simulink model "TosAeb"  (R2021a)
settings: Solver=FixedStepDiscrete  StartTime=0.0  StopTime=inf  FixedStep=0.01  RelTol=1e-3
undefined symbols (not defined in the file or in its folder): ACCModSts, AccSts1, AEB_ObjectMovingOrientation, AEB_ObjectMovingState, CN_BTCActive, CN_CurrentGearR, CN_CurrentGearR_DHT, CN_PTCActive, CN_VDCActive, CN_VSC_EspOff, CN_VSC_FCW_AEB_Error, CN_VSC_FCW_AEB_NoDispay, CN_VSC_FCW_AEB_PerfmDgdtn, CN_VSC_FCW_AEB_TempUnAvlbl, CN_VSC_VehStandstill, CNaccelAvoidMax, CNaccelAvoidMin, CNbrakePedalPress, CNcomfortStrLeftAccelMax, CNcomfortStrLeftAccelMin, CNcomfortStrRightAccelMax, CNcomfortStrRightAccelMin, CNDrvDoorOpen, CNDrvModeEco, CNDrvModeNorm, CNDrvModeOther, CNDrvModeSport, CNDrvSBRreminder, CNNull, CNobjInvalidID, CNPassDoorOpen, CNPassSBRreminder, CNPEBSAccelNegInf, CNPEBSAccelNull, CNPEBSTimeInf, CNPEBSTimeNull, CNSTOPMAX, CNSTOPNULL, CNsubjWidth, CNSwtOFF, CNSwtON, CNTTCMAX, CNTTCNULL, CNVehConfigAEBCycNone, CNVehConfigAEBNone, CNVehConfigAEBPedNone, CNVehConfigFCWNone, CNVehConfigJANone, CollisionSeverity, FALSE, FCWSnvty, JACycObjCalcInfoDefaultParams, JAPedObjCalcInfoDefaultParams, JAVehObjCalcInfoDefaultParams, latCycObjCalcInfoDefaultParams, latPedObjCalcInfoDefaultParams, longObjCalcInfoDefaultParams, ModSts, movingStatus, ObjectType ... (+1112)
libraries: simulink x3031, AEBSFunctionLib x50, Ta_Library x33, AEBSStateMachineLib x23, Mylibrary x12, simulink_extras x7, ObjSelect_lib x3, dspstat3 x2
(part 38 of 102)

== system TosAeb/FcwAebJa_Step_sys/ALG_AEBSystem/ALG_AEBController/AEB_FuncCondCalcApp/CycFunctionCondCalc/cycPrefillSuppAborCond/cycPrefillSuppCondCalc/cycPrefillSuppCondFinalJudge ==
  cycPrefillObjRelSuppCondFulfilled : Inport
  cycPrefillVehRelSuppCondFulfilled : Inport  Port=2
  cycPrefillSituationRelSuppCondFulfilled : Inport  Port=3
  Compare To Constant : simulink/Logic and Bit Operations/Compare To Constant  relop===  const=TRUE  OutDataTypeStr=boolean
  Compare To Constant1 : simulink/Logic and Bit Operations/Compare To Constant  relop===  const=TRUE  OutDataTypeStr=boolean
  Compare To Constant2 : simulink/Logic and Bit Operations/Compare To Constant  relop===  const=TRUE  OutDataTypeStr=boolean
  Logical Operator : Logic  Operator=OR  Inputs=3  AllPortsSameDT=off  OutDataTypeStr=boolean
  cycPrefillSuppCondFulfilled : Outport
  wires:
  Logical Operator/1 -> cycPrefillSuppCondFulfilled/1
  cycPrefillSituationRelSuppCondFulfilled/1 -> Compare To Constant2/1
  cycPrefillObjRelSuppCondFulfilled/1 -> Compare To Constant/1
  cycPrefillVehRelSuppCondFulfilled/1 -> Compare To Constant1/1
  Compare To Constant/1 -> Logical Operator/1
  Compare To Constant1/1 -> Logical Operator/2
  Compare To Constant2/1 -> Logical Operator/3

== system TosAeb/FcwAebJa_Step_sys/ALG_AEBSystem/ALG_AEBController/AEB_FuncCondCalcApp/CycFunctionCondCalc/cycPrefillSuppAborCond/cycPrefillSuppCondCalc/cycPrefillVehRelSuppCondCalc ==
  subjVel : Inport
  VehConfigIbooster : Inport  Port=2
  ESP_MasterCylindBrakePressValid : Inport  Port=3
  ESP_MasterCylindBrakePress : Inport  Port=4
  IB_BrkPedalAppld : Inport  Port=5
  SteerWheelAngle : Inport  Port=6
  SteerWheelSpd : Inport  Port=7
  AccrCyclPosnDiagc : Inport  Port=8
  vehStateCalcCycPrefillSuppFlg : Inport  Port=9
  Abs : Abs
  Abs1 : Abs
  AccReleventSuppCondDelayAbortion : AEBSFunctionLib/StateDelayAbortion  SystemSampleTime=-1  VariantActivationTime=update diagram  Latency=0  AutoFrameSizeCalculation=off
  Compare To Constant : simulink/Logic and Bit Operations/Compare To Constant  relop=>  const=P_AEB_Func_cycPrefillStrWheelAngleSupThres  OutDataTypeStr=boolean
  Compare To Constant1 : simulink/Logic and Bit Operations/Compare To Constant  relop=>  const=P_AEB_Func_cycPrefillGasPedalSupThres  OutDataTypeStr=boolean
  Compare To Constant2 : simulink/Logic and Bit Operations/Compare To Constant  relop=>  const=P_AEB_Func_cycPrefillStrWheelAngleSpdSupThres  OutDataTypeStr=boolean
  Compare To Constant7 : simulink/Logic and Bit Operations/Compare To Constant  relop===  const=TRUE  OutDataTypeStr=boolean
  Compare To Constant8 : simulink/Logic and Bit Operations/Compare To Constant  relop=<  const=P_AEB_Func_cycPrefillSubjVelMin  OutDataTypeStr=boolean
  Compare To Constant9 : simulink/Logic and Bit Operations/Compare To Constant  relop=>  const=P_AEB_Func_cycPrefillSubjVelMax  OutDataTypeStr=boolean
  Constant : Constant  Value=P_AEB_Func_cycPrefillStrCondAborDelayTime
  Constant1 : Constant  Value=0.02
  Constant2 : Constant  Value=P_AEB_Func_cycPrefillAccrCondAborDelayTime
  Constant3 : Constant  Value=0.02
  Logical Operator : Logic  Operator=OR  AllPortsSameDT=off  OutDataTypeStr=boolean
  Logical Operator4 : Logic  Operator=OR  AllPortsSameDT=off  OutDataTypeStr=boolean
  Logical Operator5 : Logic  Operator=OR  Inputs=5  AllPortsSameDT=off  OutDataTypeStr=boolean
  StrReleventSuppCondDelayAbortion : AEBSFunctionLib/StateDelayAbortion  SystemSampleTime=-1  VariantActivationTime=update diagram  Latency=0  AutoFrameSizeCalculation=off
  brakeReleventSuppCond : Subsystem
  cycPrefillVehRelSuppCondFulfilled : Outport
  wires:
  IB_BrkPedalAppld/1 -> brakeReleventSuppCond/4(IB_BrkPedalAppld)
  ESP_MasterCylindBrakePress/1 -> brakeReleventSuppCond/3(ESP_MasterCylindBrakePress)
  ESP_MasterCylindBrakePressValid/1 -> brakeReleventSuppCond/2(ESP_MasterCylindBrakePressValid)
  VehConfigIbooster/1 -> brakeReleventSuppCond/1(VehConfigIbooster)
  Compare To Constant1/1 -> AccReleventSuppCondDelayAbortion/1
  Constant2/1 -> AccReleventSuppCondDelayAbortion/2
  Constant3/1 -> AccReleventSuppCondDelayAbortion/3
  AccrCyclPosnDiagc/1 -> Compare To Constant1/1
  Logical Operator4/1 -> StrReleventSuppCondDelayAbortion/1
  Constant1/1 -> StrReleventSuppCondDelayAbortion/3
  Constant/1 -> StrReleventSuppCondDelayAbortion/2
  Logical Operator5/1 -> cycPrefillVehRelSuppCondFulfilled/1  "cycPrefillVehRelSuppCondFulfilled"
  subjVel/1 -> Compare To Constant9/1, Compare To Constant8/1
  Logical Operator/1 -> Logical Operator5/1  "subjVelReleventSuppCondFulfilled"
  Compare To Constant7/1 -> Logical Operator5/5  "vehStateCalcCycPrefillSuppCondFulfilled"
  Compare To Constant9/1 -> Logical Operator/2
  brakeReleventSuppCond/1(brakeReleventSuppCond) -> Logical Operator5/2  "BrkReleventSuppCondFulfilled"
  StrReleventSuppCondDelayAbortion/1 -> Logical Operator5/3  "strReleventSuppCondFulfilled"
  SteerWheelAngle/1 -> Abs/1
  Abs/1 -> Compare To Constant/1
  Compare To Constant/1 -> Logical Operator4/1
  SteerWheelSpd/1 -> Abs1/1
  Compare To Constant2/1 -> Logical Operator4/2
  Compare To Constant8/1 -> Logical Operator/1
  vehStateCalcCycPrefillSuppFlg/1 -> Compare To Constant7/1
  Abs1/1 -> Compare To Constant2/1
  AccReleventSuppCondDelayAbortion/1 -> Logical Operator5/4  "accReleventSuppCondFulfilled"

== system TosAeb/FcwAebJa_Step_sys/ALG_AEBSystem/ALG_AEBController/AEB_FuncCondCalcApp/JaCycFunctionCondCalc/JaCycAEBfullSuppAborCond/JaCycAEBfullAborCondCalc/JaCycAEBfullAborCondFinalJudge ==
  JaCycAEBfullObjRelAborCond : Inport
  JaCycAEBfullVehRelAborCond : Inport  Port=2
  Compare To Constant1 : simulink/Logic and Bit Operations/Compare To Constant  relop===  const=TRUE  OutDataTypeStr=boolean
  Compare To Constant3 : simulink/Logic and Bit Operations/Compare To Constant  relop===  const=TRUE  OutDataTypeStr=boolean
  Logical Operator : Logic  Operator=OR  AllPortsSameDT=off  OutDataTypeStr=boolean
  JaCycAEBfullAborCondNoStndstillFulfilled : Outport
  JaCycAEBfullAborCondStndstillFulfilled : Outport  Port=2
  wires:
  Compare To Constant3/1 -> Logical Operator/1
  JaCycAEBfullVehRelAborCond/1 -> Compare To Constant1/1
  Logical Operator/1 -> JaCycAEBfullAborCondNoStndstillFulfilled/1  "AEBJAPedAEBfullAborCondNoStndstillFulfilled"
  JaCycAEBfullObjRelAborCond/1 -> Compare To Constant3/1
  Compare To Constant1/1 -> Logical Operator/2, JaCycAEBfullAborCondStndstillFulfilled/1  "AEBJAPedAEBfullAborCondStndstillFulfilled"

== system TosAeb/FcwAebJa_Step_sys/ALG_AEBSystem/ALG_AEBController/AEB_FuncCondCalcApp/JaCycFunctionCondCalc/JaCycAEBfullSuppAborCond/JaCycAEBfullAborCondCalc/JaCycAEBfullObjRelAborCondCalc ==
  ObjectID : Inport
  Compare To Constant4 : simulink/Logic and Bit Operations/Compare To Constant  relop===  const=CNobjInvalidID  OutDataTypeStr=boolean
  Logical Operator : Logic  Operator=OR  AllPortsSameDT=off  OutDataTypeStr=boolean
  Relational Operator2 : RelationalOperator  Operator=~=  OutDataTypeStr=boolean  RndMeth=Simplest
  Unit Delay1 : UnitDelay  SampleTime=-1  HasFrameUpgradeWarning=on
  JaCycAEBfullObjRelAborCond : Outport
  wires:
  Logical Operator/1 -> JaCycAEBfullObjRelAborCond/1
  ObjectID/1 -> Unit Delay1/1, Relational Operator2/1, Compare To Constant4/1
  Unit Delay1/1 -> Relational Operator2/2
  Compare To Constant4/1 -> Logical Operator/1
  Relational Operator2/1 -> Logical Operator/2

== system TosAeb/FcwAebJa_Step_sys/ALG_AEBSystem/ALG_AEBController/AEB_FuncCondCalcApp/JaCycFunctionCondCalc/JaCycAEBfullSuppAborCond/JaCycAEBfullAborCondCalc/JaCycAEBfullVehRelAborCondCalc ==
  SteerWheelSpd_DegS : Inport
  AccelPedalPositionDiag : Inport  Port=2
  VSC_JaCycAEBfullAborCond : Inport  Port=3
  Abs : Abs
  Abs1 : Abs
  Compare To Constant : simulink/Logic and Bit Operations/Compare To Constant  relop=>  const=P_AEB_Func_JaCycAEBfullStrWheelAngleSpdAborThres  OutDataTypeStr=boolean
  Compare To Constant1 : simulink/Logic and Bit Operations/Compare To Constant  relop=>  const=P_AEB_Func_JaCycAEBfullGasPedalAborThres  OutDataTypeStr=boolean
  Compare To Constant3 : simulink/Logic and Bit Operations/Compare To Constant  relop===  const=TRUE  OutDataTypeStr=boolean
  Logical Operator2 : Logic  Operator=OR  Inputs=3  AllPortsSameDT=off  OutDataTypeStr=boolean
  JaCycAEBfullVehRelAborCond : Outport
  wires:
  Compare To Constant3/1 -> Logical Operator2/3
  VSC_JaCycAEBfullAborCond/1 -> Compare To Constant3/1
  Logical Operator2/1 -> JaCycAEBfullVehRelAborCond/1  "AEBJAPedAEBfullVehRelAborCondFulfilled"
  SteerWheelSpd_DegS/1 -> Abs/1
  Abs1/1 -> Compare To Constant1/1
  AccelPedalPositionDiag/1 -> Abs1/1
  Abs/1 -> Compare To Constant/1
  Compare To Constant/1 -> Logical Operator2/1
  Compare To Constant1/1 -> Logical Operator2/2

== system TosAeb/FcwAebJa_Step_sys/ALG_AEBSystem/ALG_AEBController/AEB_FuncCondCalcApp/JaCycFunctionCondCalc/JaCycAEBfullSuppAborCond/JaCycAEBfullSuppCondCalc/JaCycAEBfullSuppCondFinalJudge ==
  JaCycAEBfullObjRelActiveCond : Inport
  JaCycAEBfullVehRelActiveCond : Inport  Port=2
  JaCycAEBfullSitRelActiveCond : Inport  Port=3
  Compare To Constant : simulink/Logic and Bit Operations/Compare To Constant  relop===  const=FALSE  OutDataTypeStr=boolean
  Compare To Constant1 : simulink/Logic and Bit Operations/Compare To Constant  relop===  const=FALSE  OutDataTypeStr=boolean
  Compare To Constant2 : simulink/Logic and Bit Operations/Compare To Constant  relop===  const=FALSE  OutDataTypeStr=boolean
  Logical Operator : Logic  Operator=OR  Inputs=3  AllPortsSameDT=off  OutDataTypeStr=boolean
  JaCycAEBfullSuppCond : Outport
  wires:
  Compare To Constant2/1 -> Logical Operator/3
  Compare To Constant1/1 -> Logical Operator/2
  Compare To Constant/1 -> Logical Operator/1
  JaCycAEBfullVehRelActiveCond/1 -> Compare To Constant1/1
  JaCycAEBfullObjRelActiveCond/1 -> Compare To Constant/1
  JaCycAEBfullSitRelActiveCond/1 -> Compare To Constant2/1
  Logical Operator/1 -> JaCycAEBfullSuppCond/1

== system TosAeb/FcwAebJa_Step_sys/ALG_AEBSystem/ALG_AEBController/AEB_FuncCondCalcApp/JaCycFunctionCondCalc/JaCycAEBfullSuppAborCond/JaCycAEBfullSuppCondCalc/JaCycAEBfullSuppObjCondCalc ==
  ObjectMovingOrientation : Inport
  ObjectID : Inport  Port=2
  ObjectExistProb : Inport  Port=3
  ObjectIsObstacleProb : Inport  Port=4
  ObjectTypeCameraConfirmed : Inport  Port=5
  dy : Inport  Port=6
  vxAbsObj : Inport  Port=7
  vyRelCorr : Inport  Port=8
  ObjFusQuality : Inport  Port=9
  Abs : Abs
  Compare To Constant1 : simulink/Logic and Bit Operations/Compare To Constant  relop=>  const=P_AEB_Func_JaCycAEBfullObjVelSupThresMin  OutDataTypeStr=boolean
  Compare To Constant11 : simulink/Logic and Bit Operations/Compare To Constant  relop===  const=TRUE  OutDataTypeStr=boolean
  Compare To Constant15 : simulink/Logic and Bit Operations/Compare To Constant  relop=~=  const=CNobjInvalidID  OutDataTypeStr=boolean
  Compare To Constant16 : simulink/Logic and Bit Operations/Compare To Constant  relop=>  const=P_AEB_Func_JaCycAEBfullObjObstacleProbSupThres  OutDataTypeStr=boolean
  Compare To Constant17 : simulink/Logic and Bit Operations/Compare To Constant  relop=~=  const=CNobjInvalidID  OutDataTypeStr=boolean
  Compare To Constant18 : simulink/Logic and Bit Operations/Compare To Constant  relop===  const=TRUE  OutDataTypeStr=boolean
  Compare To Constant2 : simulink/Logic and Bit Operations/Compare To Constant  relop=<  const=P_AEB_Func_JaCycAEBfullObjVelSupThresMax  OutDataTypeStr=boolean
  Compare To Constant3 : simulink/Logic and Bit Operations/Compare To Constant  relop===  const=AEB_ObjectMovingOrientation.Crossing  OutDataTypeStr=boolean
  Compare To Constant4 : simulink/Logic and Bit Operations/Compare To Constant  relop===  const=AEB_ObjectMovingOrientation.InvDir  OutDataTypeStr=boolean
  Compare To Constant5 : simulink/Logic and Bit Operations/Compare To Constant  relop=<  const=P_AEB_Func_JaCycAEBfullObjDySupThres  OutDataTypeStr=boolean
  Logical Operator1 : Logic  AllPortsSameDT=off  OutDataTypeStr=boolean
  Logical Operator2 : Logic  Operator=OR  AllPortsSameDT=off  OutDataTypeStr=boolean
  Logical Operator4 : Logic  Inputs=7  AllPortsSameDT=off  OutDataTypeStr=boolean
  Logical Operator6 : Logic  AllPortsSameDT=off  OutDataTypeStr=boolean
  Logical Operator7 : Logic  AllPortsSameDT=off  OutDataTypeStr=boolean
  Math Function : Math  Operator=hypot  SignedPower=on  SaturateOnIntegerOverflow=on
  Product1 : Product
  Relational Operator2 : RelationalOperator  Operator===  OutDataTypeStr=boolean  RndMeth=Simplest
  Unit Delay1 : UnitDelay  SampleTime=-1  HasFrameUpgradeWarning=on
  JaCycAEBfullObjActiveCond : Outport
  wires:
  ObjectMovingOrientation/1 -> Compare To Constant4/1, Compare To Constant3/1
  Compare To Constant15/1 -> Logical Operator6/1
  Relational Operator2/1 -> Logical Operator6/2
  Unit Delay1/1 -> Relational Operator2/2
  ObjectID/1 -> Compare To Constant17/1, Unit Delay1/1, Relational Operator2/1, Compare To Constant15/1
  ObjectExistProb/1 -> Product1/1
  Product1/1 -> Compare To Constant16/1
  ObjectIsObstacleProb/1 -> Product1/2
  Compare To Constant16/1 -> Logical Operator7/2
  Compare To Constant17/1 -> Logical Operator7/1
  ObjectTypeCameraConfirmed/1 -> Compare To Constant18/1
  vxAbsObj/1 -> Math Function/1
  vyRelCorr/1 -> Math Function/2
  Math Function/1 -> Compare To Constant1/1, Compare To Constant2/1
  Compare To Constant1/1 -> Logical Operator1/1
  Compare To Constant2/1 -> Logical Operator1/2
  Logical Operator4/1 -> JaCycAEBfullObjActiveCond/1
  Compare To Constant4/1 -> Logical Operator2/1
  Compare To Constant3/1 -> Logical Operator2/2
  Logical Operator1/1 -> Logical Operator4/6  "objVelocityCondFulfilled"
  Logical Operator7/1 -> Logical Operator4/3  "objQualityCondFulfilled"
  Logical Operator6/1 -> Logical Operator4/2  "objIDCondFulfilled"
  Logical Operator2/1 -> Logical Operator4/1  "objMovingOrientationCondFulfilled"
  Compare To Constant18/1 -> Logical Operator4/4  "objFusionCondFulfilled"
  Abs/1 -> Compare To Constant5/1
  dy/1 -> Abs/1
  Compare To Constant5/1 -> Logical Operator4/5
  ObjFusQuality/1 -> Compare To Constant11/1
  Compare To Constant11/1 -> Logical Operator4/7  "ObjFusQuality"

== system TosAeb/FcwAebJa_Step_sys/ALG_AEBSystem/ALG_AEBController/AEB_FuncCondCalcApp/JaCycFunctionCondCalc/JaCycAEBfullSuppAborCond/JaCycAEBfullSuppCondCalc/JaCycAEBfullSuppSitCondCalc ==
  distBraking : Inport
  distRadical2Collsion : Inport  Port=2
  tPredictTime : Inport  Port=3
  TTB : Inport  Port=4
  ObjColliFlg : Inport  Port=5
  Add : Sum  Inputs=2
  Add1 : Sum  Inputs=2
  Compare To Constant : simulink/Logic and Bit Operations/Compare To Constant  relop===  const=TRUE  OutDataTypeStr=boolean
  Constant2 : Constant  Value=P_AEB_Func_JaCycAEBfullBrkDistSecure
  Constant3 : Constant  Value=P_AEB_Func_JaCycAEBfullTtcTimeOffset
  Logical Operator1 : Logic  AllPortsSameDT=off  OutDataTypeStr=boolean
  Relational Operator : RelationalOperator  Operator=>  OutDataTypeStr=boolean  RndMeth=Simplest
  Relational Operator1 : RelationalOperator  Operator=<  OutDataTypeStr=boolean  RndMeth=Simplest
  Terminator : Terminator
  JaCycAEBfullSuppSitActiveCond : Outport
  wires:
  TTB/1 -> Add1/1
  tPredictTime/1 -> Relational Operator1/1
  Add1/1 -> Relational Operator1/2
  Constant3/1 -> Add1/2
  Constant2/1 -> Add/2
  Relational Operator1/1 -> Logical Operator1/1
  ObjColliFlg/1 -> Compare To Constant/1
  Logical Operator1/1 -> JaCycAEBfullSuppSitActiveCond/1
  Relational Operator/1 -> Terminator/1
  Compare To Constant/1 -> Logical Operator1/2
  distBraking/1 -> Add/1
  Add/1 -> Relational Operator/1
  distRadical2Collsion/1 -> Relational Operator/2

== system TosAeb/FcwAebJa_Step_sys/ALG_AEBSystem/ALG_AEBController/AEB_FuncCondCalcApp/JaCycFunctionCondCalc/JaCycAEBfullSuppAborCond/JaCycAEBfullSuppCondCalc/JaCycAEBfullSuppVehCondCalc ==
  note: Change OR to AND
  subjVel : Inport
  VehConfigIbooster : Inport  Port=2
  ESP_MasterCylindBrakePress : Inport  Port=3
  AccrPedlPosnDiagc : Inport  Port=4
  SteerWheelAngle_Deg : Inport  Port=5
  LeftTurnSWSts : Inport  Port=6
  RightTurnSWSts : Inport  Port=7
  subjLatAccel : Inport  Port=8
  VSC_JaCycAEBfullSuppCond : Inport  Port=9
  Abs : Abs
  Abs1 : Abs
  Compare To Constant1 : simulink/Logic and Bit Operations/Compare To Constant  relop===  const=FALSE  OutDataTypeStr=boolean
  Compare To Constant3 : simulink/Logic and Bit Operations/Compare To Constant  relop=<  const=P_AEB_Func_JaCycAEBfullSubjLatAccelAbsSupThres  OutDataTypeStr=boolean
  Compare To Constant4 : simulink/Logic and Bit Operations/Compare To Constant  relop===  const=TRUE  OutDataTypeStr=boolean
  Compare To Constant5 : simulink/Logic and Bit Operations/Compare To Constant  relop===  const=FALSE  OutDataTypeStr=boolean
  Compare To Constant6 : simulink/Logic and Bit Operations/Compare To Constant  relop=>  const=P_AEB_Func_JaCycAEBfullGasPedalSupThres  OutDataTypeStr=boolean
  Compare To Constant7 : simulink/Logic and Bit Operations/Compare To Constant  relop===  const=TRUE  OutDataTypeStr=boolean
  Compare To Constant8 : simulink/Logic and Bit Operations/Compare To Constant  relop=>=  const=P_AEB_Func_JaCycAEBfullSubjVelMin  OutDataTypeStr=boolean
  Compare To Constant9 : simulink/Logic and Bit Operations/Compare To Constant  relop=<=  const=P_AEB_Func_JaCycAEBfullSubjVelMax  OutDataTypeStr=boolean
  Constant : Constant  Value=P_AEB_Func_JaCycAEBfullAccrCondAborDelayTime
  Constant1 : Constant  Value=Ts
  Constant2 : Constant  Value=P_AEB_Func_JaCycAEBfullTurnSwtStsConfig
  Constant3 : Constant  Value=TRUE
  Logical Operator : Logic  AllPortsSameDT=off  OutDataTypeStr=boolean
  Logical Operator1 : Logic  Operator=OR  AllPortsSameDT=off  OutDataTypeStr=boolean
  Logical Operator2 : Logic  AllPortsSameDT=off  OutDataTypeStr=boolean
  Logical Operator3 : Logic  Operator=OR  AllPortsSameDT=off  OutDataTypeStr=boolean
  Logical Operator5 : Logic  Inputs=6  AllPortsSameDT=off  OutDataTypeStr=boolean
  Relational Operator : RelationalOperator  OutDataTypeStr=boolean  RndMeth=Simplest
  Relational Operator1 : RelationalOperator  Operator=<=  OutDataTypeStr=boolean  RndMeth=Simplest
  StateDelayAbortion1 : AEBSFunctionLib/StateDelayAbortion  SystemSampleTime=-1  VariantActivationTime=update diagram  Latency=0  AutoFrameSizeCalculation=off
  SteerWheelThresMax : Lookup_n-D  NumberOfTableDimensions=1  BreakpointsForDimension1=P_AEB_Func_JaCycAEBfullSubjVelStrWheelAngleMaxThresX  BeginIndexSearchUsingPreviousIndexResult=on  Table=P_AEB_Func_JaCycAEBfullSubjVelStrWheelAngleMaxThresY  InternalRulePriority=Speed  RndMeth=Simplest  InputSameDT=on
  SteerWheelThresMin Table : Lookup_n-D  NumberOfTableDimensions=1  BreakpointsForDimension1=P_AEB_Func_JaCycAEBfullSubjVelStrWheelAngleMinThresX  BeginIndexSearchUsingPreviousIndexResult=on  Table=P_AEB_Func_JaCycAEBfullSubjVelStrWheelAngleMinThresY  InternalRulePriority=Speed  RndMeth=Simplest  InputSameDT=on
  Switch : Switch  Criteria=u2 ~= 0
  brakeReleventSuppCond : Subsystem
  JaCycAEBfullVehActiveCond : Outport
  wires:
  Compare To Constant1/1 -> Logical Operator5/6
  VSC_JaCycAEBfullSuppCond/1 -> Compare To Constant1/1
  StateDelayAbortion1/1 -> Logical Operator3/2  "accReleventSuppCondFulfilled"
  LeftTurnSWSts/1 -> Compare To Constant7/1
  Compare To Constant8/1 -> Logical Operator/1
  SteerWheelAngle_Deg/1 -> Abs/1
  brakeReleventSuppCond/1(brakeReleventSuppCond) -> Logical Operator3/1  "BrkReleventSuppCondFulfilled"
  Compare To Constant9/1 -> Logical Operator/2
  AccrPedlPosnDiagc/1 -> Compare To Constant6/1
  Switch/1 -> Logical Operator5/4  "TrunSWReleventActiveCondFulfilled"
  Logical Operator/1 -> Logical Operator5/1  "VelocityReleventActiveCondFulfilled"
  subjVel/1 -> Compare To Constant9/1, [subjVel], Compare To Constant8/1
  Logical Operator5/1 -> JaCycAEBfullVehActiveCond/1  "AEBJAPedAEBfullVehActiveCondFulfilled"
  Compare To Constant6/1 -> StateDelayAbortion1/1
  Constant/1 -> StateDelayAbortion1/2
  Constant1/1 -> StateDelayAbortion1/3
  SteerWheelThresMin Table/1 -> Relational Operator/2
  subjLatAccel/1 -> Abs1/1
  Abs1/1 -> Compare To Constant3/1
  Compare To Constant3/1 -> Logical Operator5/5  "LaterAccReleventActiveCondFulfilled"
  RightTurnSWSts/1 -> Compare To Constant4/1
  Compare To Constant7/1 -> Logical Operator1/1
  Compare To Constant4/1 -> Logical Operator1/2
  Abs/1 -> Relational Operator1/1, Relational Operator/1
  Relational Operator1/1 -> Logical Operator2/2
  [subjVel] -> SteerWheelThresMax/1, SteerWheelThresMin Table/1
  SteerWheelThresMax/1 -> Relational Operator1/2
  Relational Operator/1 -> Logical Operator2/1
  Logical Operator2/1 -> Logical Operator5/3  "strReleventActiveCondFulfilled"
  Logical Operator3/1 -> Compare To Constant5/1
  Compare To Constant5/1 -> Logical Operator5/2  "DriverReleventActiveCondFulfilled"
  ESP_MasterCylindBrakePress/1 -> brakeReleventSuppCond/2(ESP_MasterCylindBrakePress)
  Logical Operator1/1 -> Switch/1
  Constant2/1 -> Switch/2
  Constant3/1 -> Switch/3
  VehConfigIbooster/1 -> brakeReleventSuppCond/1(VehConfigIbooster)

== system TosAeb/FcwAebJa_Step_sys/ALG_AEBSystem/ALG_AEBController/AEB_FuncCondCalcApp/JaCycFunctionCondCalc/JaCycPrefillSuppAborCond/JaCycPrefillAborCondCalc/JaCycPrefillAborCondFinalJudge ==
  JaCycPrefillObjRelAborCond : Inport
  JaCycPrefillVehRelAborCond : Inport  Port=2
  JaCycPrefillSitRelAborCond : Inport  Port=3
  Compare To Constant1 : simulink/Logic and Bit Operations/Compare To Constant  relop===  const=TRUE  OutDataTypeStr=boolean
  Compare To Constant2 : simulink/Logic and Bit Operations/Compare To Constant  relop===  const=TRUE  OutDataTypeStr=boolean
  Compare To Constant3 : simulink/Logic and Bit Operations/Compare To Constant  relop===  const=TRUE  OutDataTypeStr=boolean
  Logical Operator : Logic  Operator=OR  Inputs=3  AllPortsSameDT=off  OutDataTypeStr=boolean
  JaCycPrefillAborCond : Outport
  wires:
  Compare To Constant2/1 -> Logical Operator/3
  Compare To Constant1/1 -> Logical Operator/2
  JaCycPrefillObjRelAborCond/1 -> Compare To Constant3/1
  Logical Operator/1 -> JaCycPrefillAborCond/1  "AEBJAPedPrefillAborCondFulfilled"
  JaCycPrefillSitRelAborCond/1 -> Compare To Constant2/1
  JaCycPrefillVehRelAborCond/1 -> Compare To Constant1/1
  Compare To Constant3/1 -> Logical Operator/1

== system TosAeb/FcwAebJa_Step_sys/ALG_AEBSystem/ALG_AEBController/AEB_FuncCondCalcApp/JaCycFunctionCondCalc/JaCycPrefillSuppAborCond/JaCycPrefillAborCondCalc/JaCycPrefillObjRelAborCondCalc ==
  ObjectID : Inport
  Compare To Constant4 : simulink/Logic and Bit Operations/Compare To Constant  relop===  const=CNobjInvalidID  OutDataTypeStr=boolean
  Logical Operator : Logic  Operator=OR  AllPortsSameDT=off  OutDataTypeStr=boolean
  Relational Operator2 : RelationalOperator  Operator=~=  OutDataTypeStr=boolean  RndMeth=Simplest
  Unit Delay1 : UnitDelay  SampleTime=-1  HasFrameUpgradeWarning=on
  JaCycPrefillObjRelAborCond : Outport
  wires:
  Relational Operator2/1 -> Logical Operator/2
  Compare To Constant4/1 -> Logical Operator/1
  Unit Delay1/1 -> Relational Operator2/2
  Logical Operator/1 -> JaCycPrefillObjRelAborCond/1
  ObjectID/1 -> Compare To Constant4/1, Relational Operator2/1, Unit Delay1/1

== system TosAeb/FcwAebJa_Step_sys/ALG_AEBSystem/ALG_AEBController/AEB_FuncCondCalcApp/JaCycFunctionCondCalc/JaCycPrefillSuppAborCond/JaCycPrefillAborCondCalc/JaCycPrefillSitRelAborCondCalc ==
  Constant : Constant  Value=FALSE
  JaCycPrefillSitRelAborCond : Outport
  wires:
  Constant/1 -> JaCycPrefillSitRelAborCond/1

== system TosAeb/FcwAebJa_Step_sys/ALG_AEBSystem/ALG_AEBController/AEB_FuncCondCalcApp/JaCycFunctionCondCalc/JaCycPrefillSuppAborCond/JaCycPrefillAborCondCalc/JaCycPrefillVehRelAborCondCalc ==
  SteerWheelSpd_DegS : Inport
  AccelPedalPositionDiag : Inport  Port=2
  VSC_JaCycPrefillAborCond : Inport  Port=3
  Abs : Abs
  Abs1 : Abs
  Compare To Constant : simulink/Logic and Bit Operations/Compare To Constant  relop=>  const=P_AEB_Func_JaCycPrefillStrWheelAngleSpdAborThres  OutDataTypeStr=boolean
  Compare To Constant1 : simulink/Logic and Bit Operations/Compare To Constant  relop=>  const=P_AEB_Func_JaCycPrefillGasPedalAborThres  OutDataTypeStr=boolean
  Compare To Constant2 : simulink/Logic and Bit Operations/Compare To Constant  relop===  const=TRUE  OutDataTypeStr=boolean
  Logical Operator2 : Logic  Operator=OR  Inputs=3  AllPortsSameDT=off  OutDataTypeStr=boolean
  JaCycPrefillVehRelAborCond : Outport
  wires:
  Compare To Constant1/1 -> Logical Operator2/2
  Compare To Constant/1 -> Logical Operator2/1
  Abs/1 -> Compare To Constant/1
  AccelPedalPositionDiag/1 -> Abs1/1
  Abs1/1 -> Compare To Constant1/1
  SteerWheelSpd_DegS/1 -> Abs/1
  Logical Operator2/1 -> JaCycPrefillVehRelAborCond/1  "AEBJAPedPrefillVehRelAborCondFulfilled"
  VSC_JaCycPrefillAborCond/1 -> Compare To Constant2/1
  Compare To Constant2/1 -> Logical Operator2/3

== system TosAeb/FcwAebJa_Step_sys/ALG_AEBSystem/ALG_AEBController/AEB_FuncCondCalcApp/JaCycFunctionCondCalc/JaCycPrefillSuppAborCond/JaCycPrefillSuppCondCalc/JaCycPrefillSuppCondFinalJudge ==
  JaCycPrefillObjActvCond : Inport
  JaCycPrefillVehActvCond : Inport  Port=2
  JaCycPrefillSitActvCond : Inport  Port=3
  Compare To Constant : simulink/Logic and Bit Operations/Compare To Constant  relop===  const=FALSE  OutDataTypeStr=boolean
  Compare To Constant1 : simulink/Logic and Bit Operations/Compare To Constant  relop===  const=FALSE  OutDataTypeStr=boolean
  Compare To Constant2 : simulink/Logic and Bit Operations/Compare To Constant  relop===  const=FALSE  OutDataTypeStr=boolean
  Logical Operator : Logic  Operator=OR  Inputs=3  AllPortsSameDT=off  OutDataTypeStr=boolean
  JaCycPrefillSuppCond : Outport
  wires:
  Logical Operator/1 -> JaCycPrefillSuppCond/1
  JaCycPrefillSitActvCond/1 -> Compare To Constant2/1
  JaCycPrefillObjActvCond/1 -> Compare To Constant/1
  JaCycPrefillVehActvCond/1 -> Compare To Constant1/1
  Compare To Constant/1 -> Logical Operator/1
  Compare To Constant1/1 -> Logical Operator/2
  Compare To Constant2/1 -> Logical Operator/3

== system TosAeb/FcwAebJa_Step_sys/ALG_AEBSystem/ALG_AEBController/AEB_FuncCondCalcApp/JaCycFunctionCondCalc/JaCycPrefillSuppAborCond/JaCycPrefillSuppCondCalc/JaCycPrefillSuppObjCondCalc ==
  ObjectMovingOrientation : Inport
  ObjectID : Inport  Port=2
  ObjectExistProb : Inport  Port=3
  ObjectIsObstacleProb : Inport  Port=4
  ObjectTypeCameraConfirmed : Inport  Port=5
  dy : Inport  Port=6
  vxAbsObj : Inport  Port=7
  vyRelCorr : Inport  Port=8
  ObjFusQuality : Inport  Port=9
  Abs : Abs
  Compare To Constant1 : simulink/Logic and Bit Operations/Compare To Constant  relop=>  const=P_AEB_Func_JaCycPrefillObjVelSupThresMin  OutDataTypeStr=boolean
  Compare To Constant11 : simulink/Logic and Bit Operations/Compare To Constant  relop===  const=TRUE  OutDataTypeStr=boolean
  Compare To Constant15 : simulink/Logic and Bit Operations/Compare To Constant  relop=~=  const=CNobjInvalidID  OutDataTypeStr=boolean
  Compare To Constant16 : simulink/Logic and Bit Operations/Compare To Constant  relop=>  const=P_AEB_Func_JaCycPrefillObjObstacleProbSupThres  OutDataTypeStr=boolean
  Compare To Constant17 : simulink/Logic and Bit Operations/Compare To Constant  relop=~=  const=CNobjInvalidID  OutDataTypeStr=boolean
  Compare To Constant18 : simulink/Logic and Bit Operations/Compare To Constant  relop===  const=TRUE  OutDataTypeStr=boolean
  Compare To Constant2 : simulink/Logic and Bit Operations/Compare To Constant  relop=<  const=P_AEB_Func_JaCycPrefillObjVelSupThresMax  OutDataTypeStr=boolean
  Compare To Constant3 : simulink/Logic and Bit Operations/Compare To Constant  relop===  const=AEB_ObjectMovingOrientation.Crossing  OutDataTypeStr=boolean
  Compare To Constant4 : simulink/Logic and Bit Operations/Compare To Constant  relop===  const=AEB_ObjectMovingOrientation.InvDir  OutDataTypeStr=boolean
  Compare To Constant5 : simulink/Logic and Bit Operations/Compare To Constant  relop=<  const=P_AEB_Func_JaCycPrefillObjDySupThres  OutDataTypeStr=boolean
  Logical Operator1 : Logic  AllPortsSameDT=off  OutDataTypeStr=boolean
  Logical Operator2 : Logic  Operator=OR  AllPortsSameDT=off  OutDataTypeStr=boolean
  Logical Operator4 : Logic  Inputs=7  AllPortsSameDT=off  OutDataTypeStr=boolean
  Logical Operator6 : Logic  AllPortsSameDT=off  OutDataTypeStr=boolean
  Logical Operator7 : Logic  AllPortsSameDT=off  OutDataTypeStr=boolean
  Math Function : Math  Operator=hypot  SignedPower=on  SaturateOnIntegerOverflow=on
  Product1 : Product
  Relational Operator2 : RelationalOperator  Operator===  OutDataTypeStr=boolean  RndMeth=Simplest
  Unit Delay1 : UnitDelay  SampleTime=-1  HasFrameUpgradeWarning=on
  JaCycPrefillSuppObjActiveCond : Outport
  wires:
  Compare To Constant16/1 -> Logical Operator7/2
  Compare To Constant17/1 -> Logical Operator7/1
  Logical Operator4/1 -> JaCycPrefillSuppObjActiveCond/1
  Compare To Constant2/1 -> Logical Operator1/2
  Compare To Constant1/1 -> Logical Operator1/1
  Math Function/1 -> Compare To Constant2/1, Compare To Constant1/1
  vyRelCorr/1 -> Math Function/2
  vxAbsObj/1 -> Math Function/1
  ObjectTypeCameraConfirmed/1 -> Compare To Constant18/1
  ObjectIsObstacleProb/1 -> Product1/2
  Product1/1 -> Compare To Constant16/1
  ObjectExistProb/1 -> Product1/1
  ObjectID/1 -> Compare To Constant15/1, Relational Operator2/1, Unit Delay1/1, Compare To Constant17/1
  Unit Delay1/1 -> Relational Operator2/2
  Relational Operator2/1 -> Logical Operator6/2
  Compare To Constant15/1 -> Logical Operator6/1
  ObjectMovingOrientation/1 -> Compare To Constant3/1, Compare To Constant4/1
  Compare To Constant4/1 -> Logical Operator2/1
  Compare To Constant3/1 -> Logical Operator2/2
  Logical Operator1/1 -> Logical Operator4/6  "objVelocityCondFulfilled"
  Logical Operator7/1 -> Logical Operator4/3  "objQualityCondFulfilled"
  Logical Operator6/1 -> Logical Operator4/2  "objIDCondFulfilled"
  Logical Operator2/1 -> Logical Operator4/1  "objMovingOrientationCondFulfilled"
  Compare To Constant18/1 -> Logical Operator4/4  "objFusionCondFulfilled"
  dy/1 -> Abs/1
  Abs/1 -> Compare To Constant5/1
  Compare To Constant5/1 -> Logical Operator4/5
  ObjFusQuality/1 -> Compare To Constant11/1
  Compare To Constant11/1 -> Logical Operator4/7  "ObjFusQuality"

== system TosAeb/FcwAebJa_Step_sys/ALG_AEBSystem/ALG_AEBController/AEB_FuncCondCalcApp/JaCycFunctionCondCalc/JaCycPrefillSuppAborCond/JaCycPrefillSuppCondCalc/JaCycPrefillSuppSitCondCalc ==
  distBraking : Inport
  distRadical2Collsion : Inport  Port=2
  tPredictTime : Inport  Port=3
  TTB : Inport  Port=4
  ObjColliFlg : Inport  Port=5
  Add : Sum  Inputs=2
  Add1 : Sum  Inputs=2
  Compare To Constant : simulink/Logic and Bit Operations/Compare To Constant  relop===  const=TRUE  OutDataTypeStr=boolean
  Constant2 : Constant  Value=P_AEB_Func_JaCycPrefillBrkDistSecure
  Constant3 : Constant  Value=P_AEB_Func_JaCycPrefillTtcTimeOffset
  Logical Operator1 : Logic  AllPortsSameDT=off  OutDataTypeStr=boolean
  Relational Operator : RelationalOperator  Operator=>  OutDataTypeStr=boolean  RndMeth=Simplest
  Relational Operator1 : RelationalOperator  Operator=<  OutDataTypeStr=boolean  RndMeth=Simplest
  Terminator : Terminator
  AEBJAVehPrefillSuppSitActiveCond : Outport
  wires:
  Compare To Constant/1 -> Logical Operator1/2
  ObjColliFlg/1 -> Compare To Constant/1
  Add1/1 -> Relational Operator1/2
  Constant3/1 -> Add1/2
  Relational Operator/1 -> Terminator/1
  Logical Operator1/1 -> AEBJAVehPrefillSuppSitActiveCond/1
  Relational Operator1/1 -> Logical Operator1/1
  Constant2/1 -> Add/2
  distBraking/1 -> Add/1
  Add/1 -> Relational Operator/1
  distRadical2Collsion/1 -> Relational Operator/2
  tPredictTime/1 -> Relational Operator1/1
  TTB/1 -> Add1/1

== system TosAeb/FcwAebJa_Step_sys/ALG_AEBSystem/ALG_AEBController/AEB_FuncCondCalcApp/JaCycFunctionCondCalc/JaCycPrefillSuppAborCond/JaCycPrefillSuppCondCalc/JaCycPrefillSuppVehCondCalc ==
  note: Change < to <=,change > to >=
  note: Chang OR to AND
  subjVel : Inport
  VehConfigIbooster : Inport  Port=2
  BrakePedalStatus_G : Inport  Port=3
  ESP_MasterCylindBrakePress : Inport  Port=4
  IB_BrkPedalAppld : Inport  Port=5
  AccrPedlPosnDiagc : Inport  Port=6
  SteerWheelAngle_Deg : Inport  Port=7
  LeftTurnSWSts : Inport  Port=8
  RightTurnSWSts : Inport  Port=9
  subjLatAccel : Inport  Port=10
  VSC_JaCycPrefillSuppCond : Inport  Port=11
  1-D Lookup Table : Lookup_n-D  NumberOfTableDimensions=1  BreakpointsForDimension1=P_AEB_Func_JaCycPrefillSubjVelStrWheelAngleMinThresX  BeginIndexSearchUsingPreviousIndexResult=on  Table=P_AEB_Func_JaCycPrefillSubjVelStrWheelAngleMinThresY  ExtrapMethod=Clip  InternalRulePriority=Speed  RndMeth=Simplest  InputSameDT=on
  1-D Lookup Table1 : Lookup_n-D  NumberOfTableDimensions=1  BreakpointsForDimension1=P_AEB_Func_JaCycPrefillSubjVelStrWheelAngleMaxThresX  BeginIndexSearchUsingPreviousIndexResult=on  Table=P_AEB_Func_JaCycPrefillSubjVelStrWheelAngleMaxThresY  ExtrapMethod=Clip  InternalRulePriority=Speed  RndMeth=Simplest  InputSameDT=on
  Abs : Abs
  Abs1 : Abs
  Compare To Constant2 : simulink/Logic and Bit Operations/Compare To Constant  relop===  const=FALSE  OutDataTypeStr=boolean
  Compare To Constant3 : simulink/Logic and Bit Operations/Compare To Constant  relop=<  const=P_AEB_Func_JaCycPrefillSubjLatAccelAbsSupThres  OutDataTypeStr=boolean
  Compare To Constant4 : simulink/Logic and Bit Operations/Compare To Constant  relop===  const=FALSE  OutDataTypeStr=boolean
  Compare To Constant5 : simulink/Logic and Bit Operations/Compare To Constant  relop===  const=TRUE  OutDataTypeStr=boolean
  Compare To Constant6 : simulink/Logic and Bit Operations/Compare To Constant  relop=>  const=P_AEB_Func_JaCycPrefillGasPedalSupThres  OutDataTypeStr=boolean
  Compare To Constant7 : simulink/Logic and Bit Operations/Compare To Constant  relop===  const=TRUE  OutDataTypeStr=boolean
  Compare To Constant8 : simulink/Logic and Bit Operations/Compare To Constant  relop=>=  const=P_AEB_Func_JaCycPrefillSubjVelMin  OutDataTypeStr=boolean
  Compare To Constant9 : simulink/Logic and Bit Operations/Compare To Constant  relop=<=  const=P_AEB_Func_JaCycPrefillSubjVelMax  OutDataTypeStr=boolean
  Constant : Constant  Value=P_AEB_Func_JaCycPrefillAccrCondAborDelayTime
  Constant1 : Constant  Value=Ts
  Constant2 : Constant  Value=P_AEB_Func_JaCycPrefillTurnSwtStsConfig
  Constant3 : Constant  Value=TRUE
  Logical Operator : Logic  AllPortsSameDT=off  OutDataTypeStr=boolean
  Logical Operator1 : Logic  Operator=OR  AllPortsSameDT=off  OutDataTypeStr=boolean
  Logical Operator2 : Logic  AllPortsSameDT=off  OutDataTypeStr=boolean
  Logical Operator4 : Logic  Operator=OR  AllPortsSameDT=off  OutDataTypeStr=boolean
  Logical Operator5 : Logic  Inputs=6  AllPortsSameDT=off  OutDataTypeStr=boolean
  Relational Operator : RelationalOperator  OutDataTypeStr=boolean  RndMeth=Simplest
  Relational Operator1 : RelationalOperator  Operator=<=  OutDataTypeStr=boolean  RndMeth=Simplest
  StateDelayAbortion : AEBSFunctionLib/StateDelayAbortion  SystemSampleTime=-1  VariantActivationTime=update diagram  Latency=0  AutoFrameSizeCalculation=off
  Switch : Switch  Criteria=u2 ~= 0
  brakeReleventSuppCond : Subsystem
  JaCycPrefillVehActiveCond : Outport
  wires:
  StateDelayAbortion/1 -> Logical Operator4/2  "accReleventSuppCondFulfilled"
  Compare To Constant6/1 -> StateDelayAbortion/1
  Abs/1 -> Relational Operator/1, Relational Operator1/1
  SteerWheelAngle_Deg/1 -> Abs/1
  Compare To Constant4/1 -> Logical Operator5/6  "VSCReleventActiveCondFulfilled"
  VSC_JaCycPrefillSuppCond/1 -> Compare To Constant4/1
  Compare To Constant3/1 -> Logical Operator5/5  "LaterAccReleventActiveCondFulfilled"
  Abs1/1 -> Compare To Constant3/1
  subjLatAccel/1 -> Abs1/1
  1-D Lookup Table/1 -> Relational Operator/2
  [subjVel] -> 1-D Lookup Table1/1, 1-D Lookup Table/1
  Constant1/1 -> StateDelayAbortion/3
  Constant/1 -> StateDelayAbortion/2
  Logical Operator5/1 -> JaCycPrefillVehActiveCond/1  "AEBJAPedPrefillVehActiveCondFulfilled"
  subjVel/1 -> [subjVel], Compare To Constant9/1, Compare To Constant8/1
  Logical Operator/1 -> Logical Operator5/1  "VelocityReleventActiveCondFulfilled"
  Switch/1 -> Logical Operator5/4  "TrunSWReleventActiveCondFulfilled"
  AccrPedlPosnDiagc/1 -> Compare To Constant6/1
  Compare To Constant9/1 -> Logical Operator/2
  brakeReleventSuppCond/1(brakeReleventSuppCond) -> Logical Operator4/1  "BrkReleventSuppCondFulfilled"
  Logical Operator2/1 -> Logical Operator5/3  "strReleventActivepCondFulfilled"
  Compare To Constant8/1 -> Logical Operator/1
  LeftTurnSWSts/1 -> Compare To Constant7/1
  RightTurnSWSts/1 -> Compare To Constant5/1
  Compare To Constant7/1 -> Logical Operator1/1
  Compare To Constant5/1 -> Logical Operator1/2
  Relational Operator/1 -> Logical Operator2/1
  1-D Lookup Table1/1 -> Relational Operator1/2
  Relational Operator1/1 -> Logical Operator2/2
  Logical Operator4/1 -> Compare To Constant2/1
  Compare To Constant2/1 -> Logical Operator5/2
  IB_BrkPedalAppld/1 -> brakeReleventSuppCond/4(IB_BrkPedalAppld)
  Constant3/1 -> Switch/3
  Constant2/1 -> Switch/2
  Logical Operator1/1 -> Switch/1
  VehConfigIbooster/1 -> brakeReleventSuppCond/1(VehConfigIbooster)
  ESP_MasterCylindBrakePress/1 -> brakeReleventSuppCond/3(ESP_MasterCylindBrakePress)
  BrakePedalStatus_G/1 -> brakeReleventSuppCond/2(BrakePedalStatus_G)

== system TosAeb/FcwAebJa_Step_sys/ALG_AEBSystem/ALG_AEBController/AEB_FuncCondCalcApp/JaPedFunctionCondCalc/AEBJAPedAEBfullSuppAborCond/AEBJAPedAEBfullAborCondCalc/AEBJAPedAEBfullAborCondFinalJudge ==
  AEBJAPedAEBfullObjRelAborCondFulfilled : Inport
  AEBJAPedAEBfullVehRelAborCondFulfilled : Inport  Port=2
  Compare To Constant1 : simulink/Logic and Bit Operations/Compare To Constant  relop===  const=TRUE  OutDataTypeStr=boolean
  Compare To Constant3 : simulink/Logic and Bit Operations/Compare To Constant  relop===  const=TRUE  OutDataTypeStr=boolean
  Logical Operator : Logic  Operator=OR  AllPortsSameDT=off  OutDataTypeStr=boolean
  AEBJAPedAEBfullAborCondNoStndstillFulfilled : Outport
  AEBJAPedAEBfullAborCondStndstillFulfilled : Outport  Port=2
  wires:
  Compare To Constant1/1 -> AEBJAPedAEBfullAborCondStndstillFulfilled/1, Logical Operator/2  "AEBJAPedAEBfullAborCondStndstillFulfilled"
  AEBJAPedAEBfullObjRelAborCondFulfilled/1 -> Compare To Constant3/1
  Logical Operator/1 -> AEBJAPedAEBfullAborCondNoStndstillFulfilled/1  "AEBJAPedAEBfullAborCondNoStndstillFulfilled"
  AEBJAPedAEBfullVehRelAborCondFulfilled/1 -> Compare To Constant1/1
  Compare To Constant3/1 -> Logical Operator/1

== system TosAeb/FcwAebJa_Step_sys/ALG_AEBSystem/ALG_AEBController/AEB_FuncCondCalcApp/JaPedFunctionCondCalc/AEBJAPedAEBfullSuppAborCond/AEBJAPedAEBfullAborCondCalc/AEBJAPedAEBfullObjRelAborCondCalc ==
  ObjectID : Inport
  Compare To Constant4 : simulink/Logic and Bit Operations/Compare To Constant  relop===  const=CNobjInvalidID  OutDataTypeStr=boolean
  Logical Operator : Logic  Operator=OR  AllPortsSameDT=off  OutDataTypeStr=boolean
  Relational Operator2 : RelationalOperator  Operator=~=  OutDataTypeStr=boolean  RndMeth=Simplest
  Unit Delay1 : UnitDelay  SampleTime=-1  HasFrameUpgradeWarning=on
  AEBJAPedAEBfullObjRelAborCondFulfilled : Outport
  wires:
  Relational Operator2/1 -> Logical Operator/2
  Compare To Constant4/1 -> Logical Operator/1
  Unit Delay1/1 -> Relational Operator2/2
  ObjectID/1 -> Compare To Constant4/1, Relational Operator2/1, Unit Delay1/1
  Logical Operator/1 -> AEBJAPedAEBfullObjRelAborCondFulfilled/1

== system TosAeb/FcwAebJa_Step_sys/ALG_AEBSystem/ALG_AEBController/AEB_FuncCondCalcApp/JaPedFunctionCondCalc/AEBJAPedAEBfullSuppAborCond/AEBJAPedAEBfullAborCondCalc/AEBJAPedAEBfullVehRelAborCondCalc ==
  SteerWheelSpd_DegS : Inport
  AccelPedalPositionDiag : Inport  Port=2
  VSC_JAPedAEBfullAborCond : Inport  Port=3
  Abs : Abs
  Abs1 : Abs
  Compare To Constant : simulink/Logic and Bit Operations/Compare To Constant  relop=>  const=P_AEB_Func_JaPedAEBfullStrWheelAngleSpdAborThres  OutDataTypeStr=boolean
  Compare To Constant1 : simulink/Logic and Bit Operations/Compare To Constant  relop=>  const=P_AEB_Func_JaPedAEBfullGasPedalAborThres  OutDataTypeStr=boolean
  Compare To Constant3 : simulink/Logic and Bit Operations/Compare To Constant  relop===  const=TRUE  OutDataTypeStr=boolean
  Logical Operator2 : Logic  Operator=OR  Inputs=3  AllPortsSameDT=off  OutDataTypeStr=boolean
  AEBJAPedAEBfullVehRelAborCondFulfilled : Outport
  wires:
  Compare To Constant1/1 -> Logical Operator2/2
  Compare To Constant/1 -> Logical Operator2/1
  Abs/1 -> Compare To Constant/1
  AccelPedalPositionDiag/1 -> Abs1/1
  Abs1/1 -> Compare To Constant1/1
  SteerWheelSpd_DegS/1 -> Abs/1
  Logical Operator2/1 -> AEBJAPedAEBfullVehRelAborCondFulfilled/1  "AEBJAPedAEBfullVehRelAborCondFulfilled"
  VSC_JAPedAEBfullAborCond/1 -> Compare To Constant3/1
  Compare To Constant3/1 -> Logical Operator2/3

== system TosAeb/FcwAebJa_Step_sys/ALG_AEBSystem/ALG_AEBController/AEB_FuncCondCalcApp/JaPedFunctionCondCalc/AEBJAPedAEBfullSuppAborCond/AEBJAPedAEBfullSuppCondCalc/AEBJAPedAEBfullSuppCondFinalJudge ==
  AEBJAPedAEBfullObjRelActiveCondFulfilled : Inport
  AEBJAPedAEBfullVehRelActiveCondFulfilled : Inport  Port=2
  AEBJAPedAEBfullSituationRelActiveCondFulfilled : Inport  Port=3
  Compare To Constant : simulink/Logic and Bit Operations/Compare To Constant  relop===  const=FALSE  OutDataTypeStr=boolean
  Compare To Constant1 : simulink/Logic and Bit Operations/Compare To Constant  relop===  const=FALSE  OutDataTypeStr=boolean
  Compare To Constant2 : simulink/Logic and Bit Operations/Compare To Constant  relop===  const=FALSE  OutDataTypeStr=boolean
  Logical Operator : Logic  Operator=OR  Inputs=3  AllPortsSameDT=off  OutDataTypeStr=boolean
  AEBJAPedAEBfullSuppCondFulfilled : Outport
  wires:
  Logical Operator/1 -> AEBJAPedAEBfullSuppCondFulfilled/1
  AEBJAPedAEBfullSituationRelActiveCondFulfilled/1 -> Compare To Constant2/1
  AEBJAPedAEBfullObjRelActiveCondFulfilled/1 -> Compare To Constant/1
  AEBJAPedAEBfullVehRelActiveCondFulfilled/1 -> Compare To Constant1/1
  Compare To Constant/1 -> Logical Operator/1
  Compare To Constant1/1 -> Logical Operator/2
  Compare To Constant2/1 -> Logical Operator/3

== system TosAeb/FcwAebJa_Step_sys/ALG_AEBSystem/ALG_AEBController/AEB_FuncCondCalcApp/JaPedFunctionCondCalc/AEBJAPedAEBfullSuppAborCond/AEBJAPedAEBfullSuppCondCalc/AEBJAPedAEBfullSuppObjCondCalcu ==
  ObjectMovingOrientation : Inport
  ObjectID : Inport  Port=2
  ObjectExistProb : Inport  Port=3
  ObjectIsObstacleProb : Inport  Port=4
  ObjectTypeCameraConfirmed : Inport  Port=5
  dy : Inport  Port=6
  vxAbsObj : Inport  Port=7
  vyRelCorr : Inport  Port=8
  ObjFusQuality : Inport  Port=9
  Abs : Abs
  Compare To Constant1 : simulink/Logic and Bit Operations/Compare To Constant  relop=>  const=P_AEB_Func_JaPedAEBfullObjVelSupThresMin  OutDataTypeStr=boolean
  Compare To Constant11 : simulink/Logic and Bit Operations/Compare To Constant  relop===  const=TRUE  OutDataTypeStr=boolean
  Compare To Constant15 : simulink/Logic and Bit Operations/Compare To Constant  relop=~=  const=CNobjInvalidID  OutDataTypeStr=boolean
  Compare To Constant16 : simulink/Logic and Bit Operations/Compare To Constant  relop=>  const=P_AEB_Func_JaPedAEBfullObjObstacleProbSupThres  OutDataTypeStr=boolean
  Compare To Constant17 : simulink/Logic and Bit Operations/Compare To Constant  relop=~=  const=CNobjInvalidID  OutDataTypeStr=boolean
  Compare To Constant18 : simulink/Logic and Bit Operations/Compare To Constant  relop===  const=TRUE  OutDataTypeStr=boolean
  Compare To Constant2 : simulink/Logic and Bit Operations/Compare To Constant  relop=<  const=P_AEB_Func_JaPedAEBfullObjVelSupThresMax  OutDataTypeStr=boolean
  Compare To Constant3 : simulink/Logic and Bit Operations/Compare To Constant  relop===  const=AEB_ObjectMovingOrientation.Crossing  OutDataTypeStr=boolean
  Compare To Constant4 : simulink/Logic and Bit Operations/Compare To Constant  relop===  const=AEB_ObjectMovingOrientation.InvDir  OutDataTypeStr=boolean
  Compare To Constant5 : simulink/Logic and Bit Operations/Compare To Constant  relop=<  const=P_AEB_Func_JaPedAEBfullObjDySupThres  OutDataTypeStr=boolean
  Logical Operator1 : Logic  AllPortsSameDT=off  OutDataTypeStr=boolean
  Logical Operator2 : Logic  Operator=OR  AllPortsSameDT=off  OutDataTypeStr=boolean
  Logical Operator4 : Logic  Inputs=7  AllPortsSameDT=off  OutDataTypeStr=boolean
  Logical Operator6 : Logic  AllPortsSameDT=off  OutDataTypeStr=boolean
  Logical Operator7 : Logic  AllPortsSameDT=off  OutDataTypeStr=boolean
  Math Function : Math  Operator=hypot  SignedPower=on  SaturateOnIntegerOverflow=on
  Product1 : Product
  Relational Operator2 : RelationalOperator  Operator===  OutDataTypeStr=boolean  RndMeth=Simplest
  Unit Delay1 : UnitDelay  SampleTime=-1  HasFrameUpgradeWarning=on
  AEBJAPedAEBfullObjActiveCondFulfilled : Outport
  wires:
  Compare To Constant11/1 -> Logical Operator4/7  "ObjFusQuality"
  ObjFusQuality/1 -> Compare To Constant11/1
  Compare To Constant5/1 -> Logical Operator4/5
  dy/1 -> Abs/1
  Abs/1 -> Compare To Constant5/1
  Compare To Constant18/1 -> Logical Operator4/4  "objFusionCondFulfilled"
  Logical Operator2/1 -> Logical Operator4/1  "objMovingOrientationCondFulfilled"
  Logical Operator6/1 -> Logical Operator4/2  "objIDCondFulfilled"
  Logical Operator7/1 -> Logical Operator4/3  "objQualityCondFulfilled"
  Logical Operator1/1 -> Logical Operator4/6  "objVelocityCondFulfilled"
  Compare To Constant3/1 -> Logical Operator2/2
  Compare To Constant4/1 -> Logical Operator2/1
  Logical Operator4/1 -> AEBJAPedAEBfullObjActiveCondFulfilled/1
  Compare To Constant2/1 -> Logical Operator1/2
  Compare To Constant1/1 -> Logical Operator1/1
  Math Function/1 -> Compare To Constant2/1, Compare To Constant1/1
  vyRelCorr/1 -> Math Function/2
  vxAbsObj/1 -> Math Function/1
  ObjectTypeCameraConfirmed/1 -> Compare To Constant18/1
  Compare To Constant17/1 -> Logical Operator7/1
  Compare To Constant16/1 -> Logical Operator7/2
  ObjectIsObstacleProb/1 -> Product1/2
  Product1/1 -> Compare To Constant16/1
  ObjectExistProb/1 -> Product1/1
  ObjectID/1 -> Compare To Constant15/1, Relational Operator2/1, Unit Delay1/1, Compare To Constant17/1
  Unit Delay1/1 -> Relational Operator2/2
  Relational Operator2/1 -> Logical Operator6/2
  Compare To Constant15/1 -> Logical Operator6/1
  ObjectMovingOrientation/1 -> Compare To Constant3/1, Compare To Constant4/1

== system TosAeb/FcwAebJa_Step_sys/ALG_AEBSystem/ALG_AEBController/AEB_FuncCondCalcApp/JaPedFunctionCondCalc/AEBJAPedAEBfullSuppAborCond/AEBJAPedAEBfullSuppCondCalc/AEBJAPedAEBfullSuppSituationCondCalc ==
  distBraking : Inport
  distRadical2Collsion : Inport  Port=2
  tPredictTime : Inport  Port=3
  TTB : Inport  Port=4
  ObjColliFlg : Inport  Port=5
  Add : Sum  Inputs=2
  Add1 : Sum  Inputs=2
  Compare To Constant : simulink/Logic and Bit Operations/Compare To Constant  relop===  const=TRUE  OutDataTypeStr=boolean
  Constant2 : Constant  Value=P_AEB_Func_JaPedAEBfullBrkDistSecure
  Constant3 : Constant  Value=P_AEB_Func_JaPedAEBfullTtcTimeOffset
  Logical Operator1 : Logic  AllPortsSameDT=off  OutDataTypeStr=boolean
  Relational Operator : RelationalOperator  Operator=>  OutDataTypeStr=boolean  RndMeth=Simplest
  Relational Operator1 : RelationalOperator  Operator=<  OutDataTypeStr=boolean  RndMeth=Simplest
  Terminator : Terminator
  AEBJAPedAEBfullSuppSituationActiveCondFulfilled : Outport
  wires:
  distRadical2Collsion/1 -> Relational Operator/2
  Add/1 -> Relational Operator/1
  distBraking/1 -> Add/1
  Compare To Constant/1 -> Logical Operator1/2
  Relational Operator/1 -> Terminator/1
  Logical Operator1/1 -> AEBJAPedAEBfullSuppSituationActiveCondFulfilled/1
  ObjColliFlg/1 -> Compare To Constant/1
  Relational Operator1/1 -> Logical Operator1/1
  Constant2/1 -> Add/2
  Constant3/1 -> Add1/2
  Add1/1 -> Relational Operator1/2
  tPredictTime/1 -> Relational Operator1/1
  TTB/1 -> Add1/1
